simulink model "os_polyphase_synth_4096c"  (R2021a) (continued)
== system os_polyphase_synth_4096c/pfb_fir == (continued)
      | if( strcmp(use_hdl,'on')),
      |     set_param(gcb, 'use_embedded','off');
      |     set_param(gcb, 'MaskVisibilities',{'on','on','on','on','on','on','on','off'});
      | else,
      |     set_param(gcb, 'MaskVisibilities',{'on','on','on','on','on','on','on','on'});
      | end
  pol1_in1_tap94 : Subsystem  AncestorBlock=casper_library_pfbs/tap  mask(tap){mult_latency=2, coeff_width=18, coeff_frac_width=17, delay=512, data_width=18, bram_latency=1, use_hdl=off, use_embedded=on}
      mask prompts: mult_latency "Multiplier Latency"; coeff_width "Coefficient Bit Width In"; coeff_frac_width "Coefficient Fractional Bits"; delay "Data/Sync Delay"; data_width "Data Bit Width In"; bram_latency "BRAM Latency"; use_hdl "Use behavioral HDL for multlipliers"; use_embedded "Use embedded multipliers"
      mask init: same as os_polyphase_synth_4096c/pfb_fir/pol1_in1_tap1
      mask Callback use_hdl:
      | use_hdl = get_param(gcb,'use_hdl');
      | if( strcmp(use_hdl,'on')),
      |     set_param(gcb, 'use_embedded','off');
      |     set_param(gcb, 'MaskVisibilities',{'on','on','on','on','on','on','on','off'});
      | else,
      |     set_param(gcb, 'MaskVisibilities',{'on','on','on','on','on','on','on','on'});
      | end
  pol1_in1_tap95 : Subsystem  AncestorBlock=casper_library_pfbs/tap  mask(tap){mult_latency=2, coeff_width=18, coeff_frac_width=17, delay=512, data_width=18, bram_latency=1, use_hdl=off, use_embedded=on}
      mask prompts: mult_latency "Multiplier Latency"; coeff_width "Coefficient Bit Width In"; coeff_frac_width "Coefficient Fractional Bits"; delay "Data/Sync Delay"; data_width "Data Bit Width In"; bram_latency "BRAM Latency"; use_hdl "Use behavioral HDL for multlipliers"; use_embedded "Use embedded multipliers"
      mask init: same as os_polyphase_synth_4096c/pfb_fir/pol1_in1_tap1
      mask Callback use_hdl:
      | use_hdl = get_param(gcb,'use_hdl');
      | if( strcmp(use_hdl,'on')),
      |     set_param(gcb, 'use_embedded','off');
      |     set_param(gcb, 'MaskVisibilities',{'on','on','on','on','on','on','on','off'});
      | else,
      |     set_param(gcb, 'MaskVisibilities',{'on','on','on','on','on','on','on','on'});
      | end
  pol1_in1_tap96 : Subsystem  AncestorBlock=casper_library_pfbs/tap  mask(tap){mult_latency=2, coeff_width=18, coeff_frac_width=17, delay=512, data_width=18, bram_latency=1, use_hdl=off, use_embedded=on}
      mask prompts: mult_latency "Multiplier Latency"; coeff_width "Coefficient Bit Width In"; coeff_frac_width "Coefficient Fractional Bits"; delay "Data/Sync Delay"; data_width "Data Bit Width In"; bram_latency "BRAM Latency"; use_hdl "Use behavioral HDL for multlipliers"; use_embedded "Use embedded multipliers"
      mask init: same as os_polyphase_synth_4096c/pfb_fir/pol1_in1_tap1
      mask Callback use_hdl:
      | use_hdl = get_param(gcb,'use_hdl');
      | if( strcmp(use_hdl,'on')),
      |     set_param(gcb, 'use_embedded','off');
      |     set_param(gcb, 'MaskVisibilities',{'on','on','on','on','on','on','on','off'});
      | else,
      |     set_param(gcb, 'MaskVisibilities',{'on','on','on','on','on','on','on','on'});
      | end
  pol1_in1_tap97 : Subsystem  AncestorBlock=casper_library_pfbs/tap  mask(tap){mult_latency=2, coeff_width=18, coeff_frac_width=17, delay=512, data_width=18, bram_latency=1, use_hdl=off, use_embedded=on}
      mask prompts: mult_latency "Multiplier Latency"; coeff_width "Coefficient Bit Width In"; coeff_frac_width "Coefficient Fractional Bits"; delay "Data/Sync Delay"; data_width "Data Bit Width In"; bram_latency "BRAM Latency"; use_hdl "Use behavioral HDL for multlipliers"; use_embedded "Use embedded multipliers"
      mask init: same as os_polyphase_synth_4096c/pfb_fir/pol1_in1_tap1
      mask Callback use_hdl:
      | use_hdl = get_param(gcb,'use_hdl');
      | if( strcmp(use_hdl,'on')),
      |     set_param(gcb, 'use_embedded','off');
      |     set_param(gcb, 'MaskVisibilities',{'on','on','on','on','on','on','on','off'});
      | else,
      |     set_param(gcb, 'MaskVisibilities',{'on','on','on','on','on','on','on','on'});
      | end
  pol1_in1_tap98 : Subsystem  AncestorBlock=casper_library_pfbs/tap  mask(tap){mult_latency=2, coeff_width=18, coeff_frac_width=17, delay=512, data_width=18, bram_latency=1, use_hdl=off, use_embedded=on}
      mask prompts: mult_latency "Multiplier Latency"; coeff_width "Coefficient Bit Width In"; coeff_frac_width "Coefficient Fractional Bits"; delay "Data/Sync Delay"; data_width "Data Bit Width In"; bram_latency "BRAM Latency"; use_hdl "Use behavioral HDL for multlipliers"; use_embedded "Use embedded multipliers"
      mask init: same as os_polyphase_synth_4096c/pfb_fir/pol1_in1_tap1
      mask Callback use_hdl:
      | use_hdl = get_param(gcb,'use_hdl');
      | if( strcmp(use_hdl,'on')),
      |     set_param(gcb, 'use_embedded','off');
      |     set_param(gcb, 'MaskVisibilities',{'on','on','on','on','on','on','on','off'});
      | else,
      |     set_param(gcb, 'MaskVisibilities',{'on','on','on','on','on','on','on','on'});
      | end
  pol1_in1_tap99 : Subsystem  AncestorBlock=casper_library_pfbs/tap  mask(tap){mult_latency=2, coeff_width=18, coeff_frac_width=17, delay=512, data_width=18, bram_latency=1, use_hdl=off, use_embedded=on}
      mask prompts: mult_latency "Multiplier Latency"; coeff_width "Coefficient Bit Width In"; coeff_frac_width "Coefficient Fractional Bits"; delay "Data/Sync Delay"; data_width "Data Bit Width In"; bram_latency "BRAM Latency"; use_hdl "Use behavioral HDL for multlipliers"; use_embedded "Use embedded multipliers"
      mask init: same as os_polyphase_synth_4096c/pfb_fir/pol1_in1_tap1
      mask Callback use_hdl:
      | use_hdl = get_param(gcb,'use_hdl');
      | if( strcmp(use_hdl,'on')),
      |     set_param(gcb, 'use_embedded','off');
      |     set_param(gcb, 'MaskVisibilities',{'on','on','on','on','on','on','on','off'});
      | else,
      |     set_param(gcb, 'MaskVisibilities',{'on','on','on','on','on','on','on','on'});
      | end
  pol1_in2_coeffs : Subsystem  AncestorBlock=casper_library_pfbs/pfb_coeff_gen  mask(pfb_coeff_gen){PFBSize=12, CoeffBitWidth=18, TotalTaps=8, CoeffDistMem=off, WindowType=hamming, bram_latency=1, n_inputs=3, nput=1, fwidth=1, debug_mode=off, fan_latency=0}
      mask prompts: PFBSize "Size of PFB: (2^? pnts)"; CoeffBitWidth "Bitwidth of Coefficients:"; TotalTaps "Total Number of Taps:"; CoeffDistMem "Implement Coeff Gen in Distributed Memory"; WindowType "Windowing Function:"; bram_latency "BRAM Latency"; n_inputs "Number of Simultaneous Inputs: (2^?)"; nput "This is input number:"; fwidth "Bin Width (normal=1)"; debug_mode "Debug mode - coeffs are a [1:pfb_size*taps] ramp."; fan_latency "Fanout latency"
      mask init: same as os_polyphase_synth_4096c/pfb_fir/pol1_in1_coeffs
      settings: AttributesFormatString=|
      | PFBSize=12, n_inputs=3,
      | taps=8, input=1
  pol1_in3_coeffs : Subsystem  AncestorBlock=casper_library_pfbs/pfb_coeff_gen  mask(pfb_coeff_gen){PFBSize=12, CoeffBitWidth=18, TotalTaps=8, CoeffDistMem=off, WindowType=hamming, bram_latency=1, n_inputs=3, nput=2, fwidth=1, debug_mode=off, fan_latency=0}
      mask prompts: PFBSize "Size of PFB: (2^? pnts)"; CoeffBitWidth "Bitwidth of Coefficients:"; TotalTaps "Total Number of Taps:"; CoeffDistMem "Implement Coeff Gen in Distributed Memory"; WindowType "Windowing Function:"; bram_latency "BRAM Latency"; n_inputs "Number of Simultaneous Inputs: (2^?)"; nput "This is input number:"; fwidth "Bin Width (normal=1)"; debug_mode "Debug mode - coeffs are a [1:pfb_size*taps] ramp."; fan_latency "Fanout latency"
      mask init: same as os_polyphase_synth_4096c/pfb_fir/pol1_in1_coeffs
      settings: AttributesFormatString=|
      | PFBSize=12, n_inputs=3,
      | taps=8, input=2
  pol1_in4_coeffs : Subsystem  AncestorBlock=casper_library_pfbs/pfb_coeff_gen  mask(pfb_coeff_gen){PFBSize=12, CoeffBitWidth=18, TotalTaps=8, CoeffDistMem=off, WindowType=hamming, bram_latency=1, n_inputs=3, nput=3, fwidth=1, debug_mode=off, fan_latency=0}
      mask prompts: PFBSize "Size of PFB: (2^? pnts)"; CoeffBitWidth "Bitwidth of Coefficients:"; TotalTaps "Total Number of Taps:"; CoeffDistMem "Implement Coeff Gen in Distributed Memory"; WindowType "Windowing Function:"; bram_latency "BRAM Latency"; n_inputs "Number of Simultaneous Inputs: (2^?)"; nput "This is input number:"; fwidth "Bin Width (normal=1)"; debug_mode "Debug mode - coeffs are a [1:pfb_size*taps] ramp."; fan_latency "Fanout latency"
      mask init: same as os_polyphase_synth_4096c/pfb_fir/pol1_in1_coeffs
      settings: AttributesFormatString=|
      | PFBSize=12, n_inputs=3,
      | taps=8, input=3
  pol1_in5_coeffs : Subsystem  AncestorBlock=casper_library_pfbs/pfb_coeff_gen  mask(pfb_coeff_gen){PFBSize=12, CoeffBitWidth=18, TotalTaps=8, CoeffDistMem=off, WindowType=hamming, bram_latency=1, n_inputs=3, nput=4, fwidth=1, debug_mode=off, fan_latency=0}
      mask prompts: PFBSize "Size of PFB: (2^? pnts)"; CoeffBitWidth "Bitwidth of Coefficients:"; TotalTaps "Total Number of Taps:"; CoeffDistMem "Implement Coeff Gen in Distributed Memory"; WindowType "Windowing Function:"; bram_latency "BRAM Latency"; n_inputs "Number of Simultaneous Inputs: (2^?)"; nput "This is input number:"; fwidth "Bin Width (normal=1)"; debug_mode "Debug mode - coeffs are a [1:pfb_size*taps] ramp."; fan_latency "Fanout latency"
      mask init: same as os_polyphase_synth_4096c/pfb_fir/pol1_in1_coeffs
      settings: AttributesFormatString=|
      | PFBSize=12, n_inputs=3,
      | taps=8, input=4
  pol1_in6_coeffs : Subsystem  AncestorBlock=casper_library_pfbs/pfb_coeff_gen  mask(pfb_coeff_gen){PFBSize=12, CoeffBitWidth=18, TotalTaps=8, CoeffDistMem=off, WindowType=hamming, bram_latency=1, n_inputs=3, nput=5, fwidth=1, debug_mode=off, fan_latency=0}
      mask prompts: PFBSize "Size of PFB: (2^? pnts)"; CoeffBitWidth "Bitwidth of Coefficients:"; TotalTaps "Total Number of Taps:"; CoeffDistMem "Implement Coeff Gen in Distributed Memory"; WindowType "Windowing Function:"; bram_latency "BRAM Latency"; n_inputs "Number of Simultaneous Inputs: (2^?)"; nput "This is input number:"; fwidth "Bin Width (normal=1)"; debug_mode "Debug mode - coeffs are a [1:pfb_size*taps] ramp."; fan_latency "Fanout latency"
      mask init: same as os_polyphase_synth_4096c/pfb_fir/pol1_in1_coeffs
      settings: AttributesFormatString=|
      | PFBSize=12, n_inputs=3,
      | taps=8, input=5
  pol1_in7_coeffs : Subsystem  AncestorBlock=casper_library_pfbs/pfb_coeff_gen  mask(pfb_coeff_gen){PFBSize=12, CoeffBitWidth=18, TotalTaps=8, CoeffDistMem=off, WindowType=hamming, bram_latency=1, n_inputs=3, nput=6, fwidth=1, debug_mode=off, fan_latency=0}
      mask prompts: PFBSize "Size of PFB: (2^? pnts)"; CoeffBitWidth "Bitwidth of Coefficients:"; TotalTaps "Total Number of Taps:"; CoeffDistMem "Implement Coeff Gen in Distributed Memory"; WindowType "Windowing Function:"; bram_latency "BRAM Latency"; n_inputs "Number of Simultaneous Inputs: (2^?)"; nput "This is input number:"; fwidth "Bin Width (normal=1)"; debug_mode "Debug mode - coeffs are a [1:pfb_size*taps] ramp."; fan_latency "Fanout latency"
      mask init: same as os_polyphase_synth_4096c/pfb_fir/pol1_in1_coeffs
      settings: AttributesFormatString=|
      | PFBSize=12, n_inputs=3,
      | taps=8, input=6
  pol1_in8_coeffs : Subsystem  AncestorBlock=casper_library_pfbs/pfb_coeff_gen  mask(pfb_coeff_gen){PFBSize=12, CoeffBitWidth=18, TotalTaps=8, CoeffDistMem=off, WindowType=hamming, bram_latency=1, n_inputs=3, nput=7, fwidth=1, debug_mode=off, fan_latency=0}
      mask prompts: PFBSize "Size of PFB: (2^? pnts)"; CoeffBitWidth "Bitwidth of Coefficients:"; TotalTaps "Total Number of Taps:"; CoeffDistMem "Implement Coeff Gen in Distributed Memory"; WindowType "Windowing Function:"; bram_latency "BRAM Latency"; n_inputs "Number of Simultaneous Inputs: (2^?)"; nput "This is input number:"; fwidth "Bin Width (normal=1)"; debug_mode "Debug mode - coeffs are a [1:pfb_size*taps] ramp."; fan_latency "Fanout latency"
      mask init: same as os_polyphase_synth_4096c/pfb_fir/pol1_in1_coeffs
      settings: AttributesFormatString=|
      | PFBSize=12, n_inputs=3,
      | taps=8, input=7
  pol2_in1_delay : xbsIndex_r4/Delay  rst=off  infoeditControl=Selection of Reset will increase slice count due to use of real FFs and instead of SRLs  en=off  latency=bram_latency+fan_latency  reg_retiming=off  dbl_ovrd=off  block_type=delay
  pol2_in2_delay : xbsIndex_r4/Delay  rst=off  infoeditControl=Selection of Reset will increase slice count due to use of real FFs and instead of SRLs  en=off  latency=bram_latency+fan_latency  reg_retiming=off  dbl_ovrd=off  block_type=delay
  pol2_in3_delay : xbsIndex_r4/Delay  rst=off  infoeditControl=Selection of Reset will increase slice count due to use of real FFs and instead of SRLs  en=off  latency=bram_latency+fan_latency  reg_retiming=off  dbl_ovrd=off  block_type=delay
  pol2_in4_delay : xbsIndex_r4/Delay  rst=off  infoeditControl=Selection of Reset will increase slice count due to use of real FFs and instead of SRLs  en=off  latency=bram_latency+fan_latency  reg_retiming=off  dbl_ovrd=off  block_type=delay
  pol2_in5_delay : xbsIndex_r4/Delay  rst=off  infoeditControl=Selection of Reset will increase slice count due to use of real FFs and instead of SRLs  en=off  latency=bram_latency+fan_latency  reg_retiming=off  dbl_ovrd=off  block_type=delay
  pol2_in6_delay : xbsIndex_r4/Delay  rst=off  infoeditControl=Selection of Reset will increase slice count due to use of real FFs and instead of SRLs  en=off  latency=bram_latency+fan_latency  reg_retiming=off  dbl_ovrd=off  block_type=delay
  pol2_in7_delay : xbsIndex_r4/Delay  rst=off  infoeditControl=Selection of Reset will increase slice count due to use of real FFs and instead of SRLs  en=off  latency=bram_latency+fan_latency  reg_retiming=off  dbl_ovrd=off  block_type=delay
  pol2_in8_delay : xbsIndex_r4/Delay  rst=off  infoeditControl=Selection of Reset will increase slice count due to use of real FFs and instead of SRLs  en=off  latency=bram_latency+fan_latency  reg_retiming=off  dbl_ovrd=off  block_type=delay
  sync_out : Outport  VectorParamsAs1DForOutWhenUnconnected=off
  pol1_out1 : Outport  Port=2  VectorParamsAs1DForOutWhenUnconnected=off
  pol1_out2 : Outport  Port=3  VectorParamsAs1DForOutWhenUnconnected=off
  pol1_out3 : Outport  Port=4
  pol1_out4 : Outport  Port=5
  pol1_out5 : Outport  Port=6
  pol1_out6 : Outport  Port=7
  pol1_out7 : Outport  Port=8
  pol1_out8 : Outport  Port=9
  pol2_out1 : Outport  Port=10
  pol2_out2 : Outport  Port=11
  pol2_out3 : Outport  Port=12
  pol2_out4 : Outport  Port=13
  pol2_out5 : Outport  Port=14
  pol2_out6 : Outport  Port=15
  pol2_out7 : Outport  Port=16
  pol2_out8 : Outport  Port=17
  wires:
  pol1_in1/1 -> pol1_in1_coeffs/1(din)
  sync/1 -> pol1_in1_coeffs/2(sync), pol1_in2_coeffs/2(sync), pol1_in4_coeffs/2(sync), pol1_in3_coeffs/2(sync), pol1_in7_coeffs/2(sync), pol1_in6_coeffs/2(sync), pol1_in5_coeffs/2(sync), pol1_in8_coeffs/2(sync)
  pol1_in1_coeffs/1(dout) -> pol1_in1_first_tap/1(din)
  pol1_in1_coeffs/2(sync_out) -> pol1_in1_first_tap15/2(sync), pol1_in1_first_tap13/2(sync), pol1_in1_first_tap12/2(sync), pol1_in1_first_tap8/2(sync), pol1_in1_first_tap9/2(sync), pol1_in1_first_tap14/2(sync), pol1_in1_first_tap11/2(sync), pol1_in1_first_tap10/2(sync), pol1_in1_first_tap7/2(sync), pol1_in1_first_tap6/2(sync), pol1_in1_first_tap5/2(sync), pol1_in1_first_tap4/2(sync), pol1_in1_first_tap3/2(sync), pol1_in1_first_tap2/2(sync), pol1_in1_first_tap1/2(sync), pol1_in1_first_tap/2(sync)
  pol1_in1_coeffs/3(coeff) -> pol1_in1_first_tap8/3(coeffs), pol1_in1_first_tap/3(coeffs)
  pol1_in1_first_tap/1(dout) -> pol1_in1_tap2/1(din)
  pol1_in1_first_tap/2(sync_out) -> pol1_in1_tap2/2(sync)
  pol1_in1_first_tap/3(coeff_out) -> pol1_in1_tap2/3(coeff)
  pol1_in1_first_tap/4(taps_out) -> pol1_in1_tap2/4(taps)
  pol1_in1_tap2/1(dout) -> pol1_in1_tap1/1(din)
  pol1_in1_tap2/2(sync_out) -> pol1_in1_tap1/2(sync)
  pol1_in1_tap2/3(coeff_out) -> pol1_in1_tap1/3(coeff)
  pol1_in1_tap2/4(taps_out) -> pol1_in1_tap1/4(taps)
  pol1_in1_tap1/1(dout) -> pol1_in1_tap3/1(din)
  pol1_in1_tap1/2(sync_out) -> pol1_in1_tap3/2(sync)
  pol1_in1_tap1/3(coeff_out) -> pol1_in1_tap3/3(coeff)
  pol1_in1_tap1/4(taps_out) -> pol1_in1_tap3/4(taps)
  pol1_in1_tap3/1(dout) -> pol1_in1_tap4/1(din)
  pol1_in1_tap3/2(sync_out) -> pol1_in1_tap4/2(sync)
  pol1_in1_tap3/3(coeff_out) -> pol1_in1_tap4/3(coeff)
  pol1_in1_tap3/4(taps_out) -> pol1_in1_tap4/4(taps)
  pol1_in1_tap4/1(dout) -> pol1_in1_tap5/1(din)
  pol1_in1_tap4/2(sync_out) -> pol1_in1_tap5/2(sync)
  pol1_in1_tap4/3(coeff_out) -> pol1_in1_tap5/3(coeff)
  pol1_in1_tap4/4(taps_out) -> pol1_in1_tap5/4(taps)
  pol1_in1_tap5/1(dout) -> pol1_in1_tap6/1(din)
  pol1_in1_tap5/2(sync_out) -> pol1_in1_tap6/2(sync)
  pol1_in1_tap5/3(coeff_out) -> pol1_in1_tap6/3(coeff)
  pol1_in1_tap5/4(taps_out) -> pol1_in1_tap6/4(taps)
  pol1_in1_tap6/1(dout) -> pol1_in1_tap7/1(din)
  pol1_in1_tap6/2(sync_out) -> pol1_in1_tap7/2(sync)
  pol1_in1_tap6/3(coeff_out) -> pol1_in1_tap7/3(coeff)
  pol1_in1_tap6/4(taps_out) -> pol1_in1_tap7/4(taps)
  pol1_in2/1 -> pol1_in2_coeffs/1(din)
  pol1_in2_coeffs/1(dout) -> pol1_in1_first_tap1/1(din)
  pol1_in2_coeffs/3(coeff) -> pol1_in1_first_tap9/3(coeffs), pol1_in1_first_tap1/3(coeffs)
  pol1_in3/1 -> pol1_in3_coeffs/1(din)
  pol1_in3_coeffs/1(dout) -> pol1_in1_first_tap2/1(din)
  pol1_in3_coeffs/3(coeff) -> pol1_in1_first_tap10/3(coeffs), pol1_in1_first_tap2/3(coeffs)
  pol1_in4/1 -> pol1_in4_coeffs/1(din)
  pol1_in4_coeffs/1(dout) -> pol1_in1_first_tap3/1(din)
  pol1_in4_coeffs/3(coeff) -> pol1_in1_first_tap11/3(coeffs), pol1_in1_first_tap3/3(coeffs)
  pol1_in5/1 -> pol1_in5_coeffs/1(din)
  pol1_in5_coeffs/1(dout) -> pol1_in1_first_tap4/1(din)
  pol1_in5_coeffs/3(coeff) -> pol1_in1_first_tap12/3(coeffs), pol1_in1_first_tap4/3(coeffs)
  pol1_in6/1 -> pol1_in6_coeffs/1(din)
  pol1_in6_coeffs/1(dout) -> pol1_in1_first_tap5/1(din)
  pol1_in6_coeffs/3(coeff) -> pol1_in1_first_tap13/3(coeffs), pol1_in1_first_tap5/3(coeffs)
  pol1_in7/1 -> pol1_in7_coeffs/1(din)
  pol1_in7_coeffs/1(dout) -> pol1_in1_first_tap6/1(din)
  pol1_in7_coeffs/3(coeff) -> pol1_in1_first_tap14/3(coeffs), pol1_in1_first_tap6/3(coeffs)
  pol1_in8/1 -> pol1_in8_coeffs/1(din)
  pol1_in8_coeffs/1(dout) -> pol1_in1_first_tap7/1(din)
  pol1_in8_coeffs/3(coeff) -> pol1_in1_first_tap15/3(coeffs), pol1_in1_first_tap7/3(coeffs)
  pol2_in1/1 -> pol2_in1_delay/1
  pol2_in1_delay/1 -> pol1_in1_first_tap8/1(din)
  pol2_in2/1 -> pol2_in2_delay/1
  pol2_in2_delay/1 -> pol1_in1_first_tap9/1(din)
  pol2_in3/1 -> pol2_in3_delay/1
  pol2_in3_delay/1 -> pol1_in1_first_tap10/1(din)
  pol2_in4/1 -> pol2_in4_delay/1
  pol2_in4_delay/1 -> pol1_in1_first_tap11/1(din)
  pol2_in5/1 -> pol2_in5_delay/1
  pol2_in5_delay/1 -> pol1_in1_first_tap12/1(din)
  pol2_in6/1 -> pol2_in6_delay/1
  pol2_in6_delay/1 -> pol1_in1_first_tap13/1(din)
  pol2_in7/1 -> pol2_in7_delay/1
  pol2_in7_delay/1 -> pol1_in1_first_tap14/1(din)
  pol2_in8/1 -> pol2_in8_delay/1
  pol2_in8_delay/1 -> pol1_in1_first_tap15/1(din)
  pol1_in1_tap7/1(sync_out) -> sync_out/1
  pol1_in1_tap7/2(taps_out) -> pol1_out1/1
  pol1_in1_tap13/4(taps_out) -> pol1_in1_tap14/4(taps)
  pol1_in1_tap13/3(coeff_out) -> pol1_in1_tap14/3(coeff)
  pol1_in1_tap13/2(sync_out) -> pol1_in1_tap14/2(sync)
  pol1_in1_tap13/1(dout) -> pol1_in1_tap14/1(din)
  pol1_in1_tap12/4(taps_out) -> pol1_in1_tap13/4(taps)
  pol1_in1_tap12/3(coeff_out) -> pol1_in1_tap13/3(coeff)
  pol1_in1_tap12/2(sync_out) -> pol1_in1_tap13/2(sync)
  pol1_in1_tap12/1(dout) -> pol1_in1_tap13/1(din)
  pol1_in1_tap11/4(taps_out) -> pol1_in1_tap12/4(taps)
  pol1_in1_tap11/3(coeff_out) -> pol1_in1_tap12/3(coeff)
  pol1_in1_tap11/2(sync_out) -> pol1_in1_tap12/2(sync)
  pol1_in1_tap11/1(dout) -> pol1_in1_tap12/1(din)
  pol1_in1_tap10/4(taps_out) -> pol1_in1_tap11/4(taps)
  pol1_in1_tap10/3(coeff_out) -> pol1_in1_tap11/3(coeff)
  pol1_in1_tap10/2(sync_out) -> pol1_in1_tap11/2(sync)
  pol1_in1_tap10/1(dout) -> pol1_in1_tap11/1(din)
  pol1_in1_tap8/4(taps_out) -> pol1_in1_tap10/4(taps)
  pol1_in1_tap8/3(coeff_out) -> pol1_in1_tap10/3(coeff)
  pol1_in1_tap8/2(sync_out) -> pol1_in1_tap10/2(sync)
  pol1_in1_tap8/1(dout) -> pol1_in1_tap10/1(din)
  pol1_in1_tap9/4(taps_out) -> pol1_in1_tap8/4(taps)
  pol1_in1_tap9/3(coeff_out) -> pol1_in1_tap8/3(coeff)
  pol1_in1_tap9/2(sync_out) -> pol1_in1_tap8/2(sync)
  pol1_in1_tap9/1(dout) -> pol1_in1_tap8/1(din)
  pol1_in1_first_tap1/4(taps_out) -> pol1_in1_tap9/4(taps)
  pol1_in1_first_tap1/3(coeff_out) -> pol1_in1_tap9/3(coeff)
  pol1_in1_first_tap1/2(sync_out) -> pol1_in1_tap9/2(sync)
  pol1_in1_first_tap1/1(dout) -> pol1_in1_tap9/1(din)
  pol1_in1_first_tap2/1(dout) -> pol1_in1_tap21/1(din)
  pol1_in1_first_tap2/2(sync_out) -> pol1_in1_tap21/2(sync)
  pol1_in1_first_tap2/3(coeff_out) -> pol1_in1_tap21/3(coeff)
  pol1_in1_first_tap2/4(taps_out) -> pol1_in1_tap21/4(taps)
  pol1_in1_tap21/1(dout) -> pol1_in1_tap20/1(din)
  pol1_in1_tap21/2(sync_out) -> pol1_in1_tap20/2(sync)
  pol1_in1_tap21/3(coeff_out) -> pol1_in1_tap20/3(coeff)
  pol1_in1_tap21/4(taps_out) -> pol1_in1_tap20/4(taps)
  pol1_in1_tap20/1(dout) -> pol1_in1_tap15/1(din)
  pol1_in1_tap20/2(sync_out) -> pol1_in1_tap15/2(sync)
  pol1_in1_tap20/3(coeff_out) -> pol1_in1_tap15/3(coeff)
  pol1_in1_tap20/4(taps_out) -> pol1_in1_tap15/4(taps)
  pol1_in1_tap15/1(dout) -> pol1_in1_tap16/1(din)
  pol1_in1_tap15/2(sync_out) -> pol1_in1_tap16/2(sync)
  pol1_in1_tap15/3(coeff_out) -> pol1_in1_tap16/3(coeff)
  pol1_in1_tap15/4(taps_out) -> pol1_in1_tap16/4(taps)
  pol1_in1_tap16/1(dout) -> pol1_in1_tap17/1(din)
  pol1_in1_tap16/2(sync_out) -> pol1_in1_tap17/2(sync)
  pol1_in1_tap16/3(coeff_out) -> pol1_in1_tap17/3(coeff)
  pol1_in1_tap16/4(taps_out) -> pol1_in1_tap17/4(taps)
  pol1_in1_tap17/1(dout) -> pol1_in1_tap18/1(din)
  pol1_in1_tap17/2(sync_out) -> pol1_in1_tap18/2(sync)
  pol1_in1_tap17/3(coeff_out) -> pol1_in1_tap18/3(coeff)
  pol1_in1_tap17/4(taps_out) -> pol1_in1_tap18/4(taps)
  pol1_in1_tap18/1(dout) -> pol1_in1_tap19/1(din)
  pol1_in1_tap18/2(sync_out) -> pol1_in1_tap19/2(sync)
  pol1_in1_tap18/3(coeff_out) -> pol1_in1_tap19/3(coeff)
  pol1_in1_tap18/4(taps_out) -> pol1_in1_tap19/4(taps)
  pol1_in1_tap25/4(taps_out) -> pol1_in1_tap26/4(taps)
  pol1_in1_tap25/3(coeff_out) -> pol1_in1_tap26/3(coeff)
  pol1_in1_tap25/2(sync_out) -> pol1_in1_tap26/2(sync)
  pol1_in1_tap25/1(dout) -> pol1_in1_tap26/1(din)
  pol1_in1_tap24/4(taps_out) -> pol1_in1_tap25/4(taps)
  pol1_in1_tap24/3(coeff_out) -> pol1_in1_tap25/3(coeff)
  pol1_in1_tap24/2(sync_out) -> pol1_in1_tap25/2(sync)
  pol1_in1_tap24/1(dout) -> pol1_in1_tap25/1(din)
  pol1_in1_tap23/4(taps_out) -> pol1_in1_tap24/4(taps)
  pol1_in1_tap23/3(coeff_out) -> pol1_in1_tap24/3(coeff)
  pol1_in1_tap23/2(sync_out) -> pol1_in1_tap24/2(sync)
  pol1_in1_tap23/1(dout) -> pol1_in1_tap24/1(din)
  pol1_in1_tap22/4(taps_out) -> pol1_in1_tap23/4(taps)
  pol1_in1_tap22/3(coeff_out) -> pol1_in1_tap23/3(coeff)
  pol1_in1_tap22/2(sync_out) -> pol1_in1_tap23/2(sync)
  pol1_in1_tap22/1(dout) -> pol1_in1_tap23/1(din)
  pol1_in1_tap27/4(taps_out) -> pol1_in1_tap22/4(taps)
  pol1_in1_tap27/3(coeff_out) -> pol1_in1_tap22/3(coeff)
  pol1_in1_tap27/2(sync_out) -> pol1_in1_tap22/2(sync)
  pol1_in1_tap27/1(dout) -> pol1_in1_tap22/1(din)
  pol1_in1_tap28/4(taps_out) -> pol1_in1_tap27/4(taps)
  pol1_in1_tap28/3(coeff_out) -> pol1_in1_tap27/3(coeff)
  pol1_in1_tap28/2(sync_out) -> pol1_in1_tap27/2(sync)
  pol1_in1_tap28/1(dout) -> pol1_in1_tap27/1(din)
  pol1_in1_first_tap3/4(taps_out) -> pol1_in1_tap28/4(taps)
  pol1_in1_first_tap3/3(coeff_out) -> pol1_in1_tap28/3(coeff)
  pol1_in1_first_tap3/2(sync_out) -> pol1_in1_tap28/2(sync)
  pol1_in1_first_tap3/1(dout) -> pol1_in1_tap28/1(din)
  pol1_in1_first_tap7/1(dout) -> pol1_in1_tap49/1(din)
  pol1_in1_first_tap7/2(sync_out) -> pol1_in1_tap49/2(sync)
  pol1_in1_first_tap7/3(coeff_out) -> pol1_in1_tap49/3(coeff)
  pol1_in1_first_tap7/4(taps_out) -> pol1_in1_tap49/4(taps)
  pol1_in1_tap49/1(dout) -> pol1_in1_tap48/1(din)
  pol1_in1_tap49/2(sync_out) -> pol1_in1_tap48/2(sync)
  pol1_in1_tap49/3(coeff_out) -> pol1_in1_tap48/3(coeff)
  pol1_in1_tap49/4(taps_out) -> pol1_in1_tap48/4(taps)
  pol1_in1_tap48/1(dout) -> pol1_in1_tap43/1(din)
  pol1_in1_tap48/2(sync_out) -> pol1_in1_tap43/2(sync)
  pol1_in1_tap48/3(coeff_out) -> pol1_in1_tap43/3(coeff)
  pol1_in1_tap48/4(taps_out) -> pol1_in1_tap43/4(taps)
  pol1_in1_tap43/1(dout) -> pol1_in1_tap44/1(din)
  pol1_in1_tap43/2(sync_out) -> pol1_in1_tap44/2(sync)
  pol1_in1_tap43/3(coeff_out) -> pol1_in1_tap44/3(coeff)
  pol1_in1_tap43/4(taps_out) -> pol1_in1_tap44/4(taps)
  pol1_in1_tap44/1(dout) -> pol1_in1_tap45/1(din)
  pol1_in1_tap44/2(sync_out) -> pol1_in1_tap45/2(sync)
  pol1_in1_tap44/3(coeff_out) -> pol1_in1_tap45/3(coeff)
  pol1_in1_tap44/4(taps_out) -> pol1_in1_tap45/4(taps)
  pol1_in1_tap45/1(dout) -> pol1_in1_tap46/1(din)
  pol1_in1_tap45/2(sync_out) -> pol1_in1_tap46/2(sync)
  pol1_in1_tap45/3(coeff_out) -> pol1_in1_tap46/3(coeff)
  pol1_in1_tap45/4(taps_out) -> pol1_in1_tap46/4(taps)
  pol1_in1_tap46/1(dout) -> pol1_in1_tap47/1(din)
  pol1_in1_tap46/2(sync_out) -> pol1_in1_tap47/2(sync)
  pol1_in1_tap46/3(coeff_out) -> pol1_in1_tap47/3(coeff)
  pol1_in1_tap46/4(taps_out) -> pol1_in1_tap47/4(taps)
  pol1_in1_tap38/4(taps_out) -> pol1_in1_tap39/4(taps)
  pol1_in1_tap38/3(coeff_out) -> pol1_in1_tap39/3(coeff)
  pol1_in1_tap38/2(sync_out) -> pol1_in1_tap39/2(sync)
  pol1_in1_tap38/1(dout) -> pol1_in1_tap39/1(din)
  pol1_in1_tap37/4(taps_out) -> pol1_in1_tap38/4(taps)
  pol1_in1_tap37/3(coeff_out) -> pol1_in1_tap38/3(coeff)
  pol1_in1_tap37/2(sync_out) -> pol1_in1_tap38/2(sync)
  pol1_in1_tap37/1(dout) -> pol1_in1_tap38/1(din)
  pol1_in1_tap36/4(taps_out) -> pol1_in1_tap37/4(taps)
  pol1_in1_tap36/3(coeff_out) -> pol1_in1_tap37/3(coeff)
  pol1_in1_tap36/2(sync_out) -> pol1_in1_tap37/2(sync)
  pol1_in1_tap36/1(dout) -> pol1_in1_tap37/1(din)
  pol1_in1_tap35/4(taps_out) -> pol1_in1_tap36/4(taps)
  pol1_in1_tap35/3(coeff_out) -> pol1_in1_tap36/3(coeff)
  pol1_in1_tap35/2(sync_out) -> pol1_in1_tap36/2(sync)
  pol1_in1_tap35/1(dout) -> pol1_in1_tap36/1(din)
  pol1_in1_tap41/4(taps_out) -> pol1_in1_tap35/4(taps)
  pol1_in1_tap41/3(coeff_out) -> pol1_in1_tap35/3(coeff)
  pol1_in1_tap41/2(sync_out) -> pol1_in1_tap35/2(sync)
  pol1_in1_tap41/1(dout) -> pol1_in1_tap35/1(din)
  pol1_in1_tap42/4(taps_out) -> pol1_in1_tap41/4(taps)
  pol1_in1_tap42/3(coeff_out) -> pol1_in1_tap41/3(coeff)
  pol1_in1_tap42/2(sync_out) -> pol1_in1_tap41/2(sync)
  pol1_in1_tap42/1(dout) -> pol1_in1_tap41/1(din)
  pol1_in1_first_tap6/4(taps_out) -> pol1_in1_tap42/4(taps)
  pol1_in1_first_tap6/3(coeff_out) -> pol1_in1_tap42/3(coeff)
  pol1_in1_first_tap6/2(sync_out) -> pol1_in1_tap42/2(sync)
  pol1_in1_first_tap6/1(dout) -> pol1_in1_tap42/1(din)
  pol1_in1_first_tap5/1(dout) -> pol1_in1_tap56/1(din)
  pol1_in1_first_tap5/2(sync_out) -> pol1_in1_tap56/2(sync)
  pol1_in1_first_tap5/3(coeff_out) -> pol1_in1_tap56/3(coeff)
  pol1_in1_first_tap5/4(taps_out) -> pol1_in1_tap56/4(taps)
  pol1_in1_tap56/1(dout) -> pol1_in1_tap55/1(din)
  pol1_in1_tap56/2(sync_out) -> pol1_in1_tap55/2(sync)
  pol1_in1_tap56/3(coeff_out) -> pol1_in1_tap55/3(coeff)
  pol1_in1_tap56/4(taps_out) -> pol1_in1_tap55/4(taps)
  pol1_in1_tap55/1(dout) -> pol1_in1_tap30/1(din)
  pol1_in1_tap55/2(sync_out) -> pol1_in1_tap30/2(sync)
  pol1_in1_tap55/3(coeff_out) -> pol1_in1_tap30/3(coeff)
  pol1_in1_tap55/4(taps_out) -> pol1_in1_tap30/4(taps)
  pol1_in1_tap30/1(dout) -> pol1_in1_tap31/1(din)
  pol1_in1_tap30/2(sync_out) -> pol1_in1_tap31/2(sync)
  pol1_in1_tap30/3(coeff_out) -> pol1_in1_tap31/3(coeff)
  pol1_in1_tap30/4(taps_out) -> pol1_in1_tap31/4(taps)
  pol1_in1_tap31/1(dout) -> pol1_in1_tap32/1(din)
  pol1_in1_tap31/2(sync_out) -> pol1_in1_tap32/2(sync)
  pol1_in1_tap31/3(coeff_out) -> pol1_in1_tap32/3(coeff)
  pol1_in1_tap31/4(taps_out) -> pol1_in1_tap32/4(taps)
  pol1_in1_tap32/1(dout) -> pol1_in1_tap33/1(din)
  pol1_in1_tap32/2(sync_out) -> pol1_in1_tap33/2(sync)
  pol1_in1_tap32/3(coeff_out) -> pol1_in1_tap33/3(coeff)
  pol1_in1_tap32/4(taps_out) -> pol1_in1_tap33/4(taps)
  pol1_in1_tap33/1(dout) -> pol1_in1_tap34/1(din)
  pol1_in1_tap33/2(sync_out) -> pol1_in1_tap34/2(sync)
  pol1_in1_tap33/3(coeff_out) -> pol1_in1_tap34/3(coeff)
  pol1_in1_tap33/4(taps_out) -> pol1_in1_tap34/4(taps)
  pol1_in1_tap53/4(taps_out) -> pol1_in1_tap54/4(taps)
  pol1_in1_tap53/3(coeff_out) -> pol1_in1_tap54/3(coeff)
  pol1_in1_tap53/2(sync_out) -> pol1_in1_tap54/2(sync)
  pol1_in1_tap53/1(dout) -> pol1_in1_tap54/1(din)
  pol1_in1_tap52/4(taps_out) -> pol1_in1_tap53/4(taps)
  pol1_in1_tap52/3(coeff_out) -> pol1_in1_tap53/3(coeff)
  pol1_in1_tap52/2(sync_out) -> pol1_in1_tap53/2(sync)
  pol1_in1_tap52/1(dout) -> pol1_in1_tap53/1(din)
  pol1_in1_tap51/4(taps_out) -> pol1_in1_tap52/4(taps)
  pol1_in1_tap51/3(coeff_out) -> pol1_in1_tap52/3(coeff)
  pol1_in1_tap51/2(sync_out) -> pol1_in1_tap52/2(sync)
  pol1_in1_tap51/1(dout) -> pol1_in1_tap52/1(din)
  pol1_in1_tap50/4(taps_out) -> pol1_in1_tap51/4(taps)
  pol1_in1_tap50/3(coeff_out) -> pol1_in1_tap51/3(coeff)
  pol1_in1_tap50/2(sync_out) -> pol1_in1_tap51/2(sync)
  pol1_in1_tap50/1(dout) -> pol1_in1_tap51/1(din)
  pol1_in1_tap29/4(taps_out) -> pol1_in1_tap50/4(taps)
  pol1_in1_tap29/3(coeff_out) -> pol1_in1_tap50/3(coeff)
  pol1_in1_tap29/2(sync_out) -> pol1_in1_tap50/2(sync)
  pol1_in1_tap29/1(dout) -> pol1_in1_tap50/1(din)
  pol1_in1_tap40/4(taps_out) -> pol1_in1_tap29/4(taps)
  pol1_in1_tap40/3(coeff_out) -> pol1_in1_tap29/3(coeff)
  pol1_in1_tap40/2(sync_out) -> pol1_in1_tap29/2(sync)
  pol1_in1_tap40/1(dout) -> pol1_in1_tap29/1(din)
  pol1_in1_first_tap4/4(taps_out) -> pol1_in1_tap40/4(taps)
  pol1_in1_first_tap4/3(coeff_out) -> pol1_in1_tap40/3(coeff)
  pol1_in1_first_tap4/2(sync_out) -> pol1_in1_tap40/2(sync)
  pol1_in1_first_tap4/1(dout) -> pol1_in1_tap40/1(din)
  pol1_in1_first_tap12/1(dout) -> pol1_in1_tap91/1(din)
  pol1_in1_first_tap12/2(sync_out) -> pol1_in1_tap91/2(sync)
  pol1_in1_first_tap12/3(coeff_out) -> pol1_in1_tap91/3(coeff)
  pol1_in1_first_tap12/4(taps_out) -> pol1_in1_tap91/4(taps)
  pol1_in1_tap91/1(dout) -> pol1_in1_tap78/1(din)
  pol1_in1_tap91/2(sync_out) -> pol1_in1_tap78/2(sync)
  pol1_in1_tap91/3(coeff_out) -> pol1_in1_tap78/3(coeff)
  pol1_in1_tap91/4(taps_out) -> pol1_in1_tap78/4(taps)
  pol1_in1_tap78/1(dout) -> pol1_in1_tap102/1(din)
  pol1_in1_tap78/2(sync_out) -> pol1_in1_tap102/2(sync)
  pol1_in1_tap78/3(coeff_out) -> pol1_in1_tap102/3(coeff)
  pol1_in1_tap78/4(taps_out) -> pol1_in1_tap102/4(taps)
  pol1_in1_tap102/1(dout) -> pol1_in1_tap103/1(din)
  pol1_in1_tap102/2(sync_out) -> pol1_in1_tap103/2(sync)
  pol1_in1_tap102/3(coeff_out) -> pol1_in1_tap103/3(coeff)
  pol1_in1_tap102/4(taps_out) -> pol1_in1_tap103/4(taps)
  pol1_in1_tap103/1(dout) -> pol1_in1_tap104/1(din)
  pol1_in1_tap103/2(sync_out) -> pol1_in1_tap104/2(sync)
  pol1_in1_tap103/3(coeff_out) -> pol1_in1_tap104/3(coeff)
  pol1_in1_tap103/4(taps_out) -> pol1_in1_tap104/4(taps)
  pol1_in1_tap104/1(dout) -> pol1_in1_tap105/1(din)
  pol1_in1_tap104/2(sync_out) -> pol1_in1_tap105/2(sync)
  pol1_in1_tap104/3(coeff_out) -> pol1_in1_tap105/3(coeff)
  pol1_in1_tap104/4(taps_out) -> pol1_in1_tap105/4(taps)
  pol1_in1_tap105/1(dout) -> pol1_in1_tap106/1(din)
  pol1_in1_tap105/2(sync_out) -> pol1_in1_tap106/2(sync)
  pol1_in1_tap105/3(coeff_out) -> pol1_in1_tap106/3(coeff)
  pol1_in1_tap105/4(taps_out) -> pol1_in1_tap106/4(taps)
  pol1_in1_tap83/4(taps_out) -> pol1_in1_tap84/4(taps)
  pol1_in1_tap83/3(coeff_out) -> pol1_in1_tap84/3(coeff)
  pol1_in1_tap83/2(sync_out) -> pol1_in1_tap84/2(sync)
  pol1_in1_tap83/1(dout) -> pol1_in1_tap84/1(din)
  pol1_in1_tap82/4(taps_out) -> pol1_in1_tap83/4(taps)
  pol1_in1_tap82/3(coeff_out) -> pol1_in1_tap83/3(coeff)
  pol1_in1_tap82/2(sync_out) -> pol1_in1_tap83/2(sync)
  pol1_in1_tap82/1(dout) -> pol1_in1_tap83/1(din)
  pol1_in1_tap81/4(taps_out) -> pol1_in1_tap82/4(taps)
  pol1_in1_tap81/3(coeff_out) -> pol1_in1_tap82/3(coeff)
  pol1_in1_tap81/2(sync_out) -> pol1_in1_tap82/2(sync)
  pol1_in1_tap81/1(dout) -> pol1_in1_tap82/1(din)
  pol1_in1_tap80/4(taps_out) -> pol1_in1_tap81/4(taps)
  pol1_in1_tap80/3(coeff_out) -> pol1_in1_tap81/3(coeff)
  pol1_in1_tap80/2(sync_out) -> pol1_in1_tap81/2(sync)
  pol1_in1_tap80/1(dout) -> pol1_in1_tap81/1(din)
  pol1_in1_tap107/4(taps_out) -> pol1_in1_tap80/4(taps)
  pol1_in1_tap107/3(coeff_out) -> pol1_in1_tap80/3(coeff)
  pol1_in1_tap107/2(sync_out) -> pol1_in1_tap80/2(sync)
  pol1_in1_tap107/1(dout) -> pol1_in1_tap80/1(din)
  pol1_in1_tap108/4(taps_out) -> pol1_in1_tap107/4(taps)
  pol1_in1_tap108/3(coeff_out) -> pol1_in1_tap107/3(coeff)
  pol1_in1_tap108/2(sync_out) -> pol1_in1_tap107/2(sync)
  pol1_in1_tap108/1(dout) -> pol1_in1_tap107/1(din)
  pol1_in1_first_tap13/4(taps_out) -> pol1_in1_tap108/4(taps)
  pol1_in1_first_tap13/3(coeff_out) -> pol1_in1_tap108/3(coeff)
  pol1_in1_first_tap13/2(sync_out) -> pol1_in1_tap108/2(sync)
  pol1_in1_first_tap13/1(dout) -> pol1_in1_tap108/1(din)
  pol1_in1_first_tap14/1(dout) -> pol1_in1_tap93/1(din)
  pol1_in1_first_tap14/2(sync_out) -> pol1_in1_tap93/2(sync)
  pol1_in1_first_tap14/3(coeff_out) -> pol1_in1_tap93/3(coeff)
  pol1_in1_first_tap14/4(taps_out) -> pol1_in1_tap93/4(taps)
  pol1_in1_tap93/1(dout) -> pol1_in1_tap92/1(din)
  pol1_in1_tap93/2(sync_out) -> pol1_in1_tap92/2(sync)
  pol1_in1_tap93/3(coeff_out) -> pol1_in1_tap92/3(coeff)
  pol1_in1_tap93/4(taps_out) -> pol1_in1_tap92/4(taps)
  pol1_in1_tap92/1(dout) -> pol1_in1_tap85/1(din)
  pol1_in1_tap92/2(sync_out) -> pol1_in1_tap85/2(sync)
  pol1_in1_tap92/3(coeff_out) -> pol1_in1_tap85/3(coeff)
  pol1_in1_tap92/4(taps_out) -> pol1_in1_tap85/4(taps)
  pol1_in1_tap85/1(dout) -> pol1_in1_tap86/1(din)
  pol1_in1_tap85/2(sync_out) -> pol1_in1_tap86/2(sync)
  pol1_in1_tap85/3(coeff_out) -> pol1_in1_tap86/3(coeff)
  pol1_in1_tap85/4(taps_out) -> pol1_in1_tap86/4(taps)
  pol1_in1_tap86/1(dout) -> pol1_in1_tap87/1(din)
  pol1_in1_tap86/2(sync_out) -> pol1_in1_tap87/2(sync)
  pol1_in1_tap86/3(coeff_out) -> pol1_in1_tap87/3(coeff)
  pol1_in1_tap86/4(taps_out) -> pol1_in1_tap87/4(taps)
  pol1_in1_tap87/1(dout) -> pol1_in1_tap88/1(din)
  pol1_in1_tap87/2(sync_out) -> pol1_in1_tap88/2(sync)
  pol1_in1_tap87/3(coeff_out) -> pol1_in1_tap88/3(coeff)
  pol1_in1_tap87/4(taps_out) -> pol1_in1_tap88/4(taps)
  pol1_in1_tap88/1(dout) -> pol1_in1_tap89/1(din)
  pol1_in1_tap88/2(sync_out) -> pol1_in1_tap89/2(sync)
  pol1_in1_tap88/3(coeff_out) -> pol1_in1_tap89/3(coeff)
  pol1_in1_tap88/4(taps_out) -> pol1_in1_tap89/4(taps)
  pol1_in1_tap97/4(taps_out) -> pol1_in1_tap98/4(taps)
  pol1_in1_tap97/3(coeff_out) -> pol1_in1_tap98/3(coeff)
  pol1_in1_tap97/2(sync_out) -> pol1_in1_tap98/2(sync)
  pol1_in1_tap97/1(dout) -> pol1_in1_tap98/1(din)
  pol1_in1_tap96/4(taps_out) -> pol1_in1_tap97/4(taps)
  pol1_in1_tap96/3(coeff_out) -> pol1_in1_tap97/3(coeff)
  pol1_in1_tap96/2(sync_out) -> pol1_in1_tap97/2(sync)
  pol1_in1_tap96/1(dout) -> pol1_in1_tap97/1(din)
  pol1_in1_tap95/4(taps_out) -> pol1_in1_tap96/4(taps)
  pol1_in1_tap95/3(coeff_out) -> pol1_in1_tap96/3(coeff)
  pol1_in1_tap95/2(sync_out) -> pol1_in1_tap96/2(sync)
  pol1_in1_tap95/1(dout) -> pol1_in1_tap96/1(din)
  pol1_in1_tap94/4(taps_out) -> pol1_in1_tap95/4(taps)
  pol1_in1_tap94/3(coeff_out) -> pol1_in1_tap95/3(coeff)
  pol1_in1_tap94/2(sync_out) -> pol1_in1_tap95/2(sync)
  pol1_in1_tap94/1(dout) -> pol1_in1_tap95/1(din)
  pol1_in1_tap99/4(taps_out) -> pol1_in1_tap94/4(taps)
  pol1_in1_tap99/3(coeff_out) -> pol1_in1_tap94/3(coeff)
  pol1_in1_tap99/2(sync_out) -> pol1_in1_tap94/2(sync)
  pol1_in1_tap99/1(dout) -> pol1_in1_tap94/1(din)
  pol1_in1_tap100/4(taps_out) -> pol1_in1_tap99/4(taps)
  pol1_in1_tap100/3(coeff_out) -> pol1_in1_tap99/3(coeff)
  pol1_in1_tap100/2(sync_out) -> pol1_in1_tap99/2(sync)
  pol1_in1_tap100/1(dout) -> pol1_in1_tap99/1(din)
  pol1_in1_first_tap15/4(taps_out) -> pol1_in1_tap100/4(taps)
  pol1_in1_first_tap15/3(coeff_out) -> pol1_in1_tap100/3(coeff)
  pol1_in1_first_tap15/2(sync_out) -> pol1_in1_tap100/2(sync)
  pol1_in1_first_tap15/1(dout) -> pol1_in1_tap100/1(din)
  pol1_in1_first_tap11/1(dout) -> pol1_in1_tap77/1(din)
  pol1_in1_first_tap11/2(sync_out) -> pol1_in1_tap77/2(sync)
  pol1_in1_first_tap11/3(coeff_out) -> pol1_in1_tap77/3(coeff)
  pol1_in1_first_tap11/4(taps_out) -> pol1_in1_tap77/4(taps)
  pol1_in1_tap77/1(dout) -> pol1_in1_tap76/1(din)
  pol1_in1_tap77/2(sync_out) -> pol1_in1_tap76/2(sync)
  pol1_in1_tap77/3(coeff_out) -> pol1_in1_tap76/3(coeff)
  pol1_in1_tap77/4(taps_out) -> pol1_in1_tap76/4(taps)
  pol1_in1_tap76/1(dout) -> pol1_in1_tap71/1(din)
  pol1_in1_tap76/2(sync_out) -> pol1_in1_tap71/2(sync)
  pol1_in1_tap76/3(coeff_out) -> pol1_in1_tap71/3(coeff)
  pol1_in1_tap76/4(taps_out) -> pol1_in1_tap71/4(taps)
  pol1_in1_tap71/1(dout) -> pol1_in1_tap72/1(din)
  pol1_in1_tap71/2(sync_out) -> pol1_in1_tap72/2(sync)
  pol1_in1_tap71/3(coeff_out) -> pol1_in1_tap72/3(coeff)
  pol1_in1_tap71/4(taps_out) -> pol1_in1_tap72/4(taps)
  pol1_in1_tap72/1(dout) -> pol1_in1_tap73/1(din)
  pol1_in1_tap72/2(sync_out) -> pol1_in1_tap73/2(sync)
  pol1_in1_tap72/3(coeff_out) -> pol1_in1_tap73/3(coeff)
  pol1_in1_tap72/4(taps_out) -> pol1_in1_tap73/4(taps)
  pol1_in1_tap73/1(dout) -> pol1_in1_tap74/1(din)
  pol1_in1_tap73/2(sync_out) -> pol1_in1_tap74/2(sync)
  pol1_in1_tap73/3(coeff_out) -> pol1_in1_tap74/3(coeff)
  pol1_in1_tap73/4(taps_out) -> pol1_in1_tap74/4(taps)
  pol1_in1_tap74/1(dout) -> pol1_in1_tap75/1(din)
  pol1_in1_tap74/2(sync_out) -> pol1_in1_tap75/2(sync)
  pol1_in1_tap74/3(coeff_out) -> pol1_in1_tap75/3(coeff)
  pol1_in1_tap74/4(taps_out) -> pol1_in1_tap75/4(taps)
  pol1_in1_tap66/4(taps_out) -> pol1_in1_tap67/4(taps)
  pol1_in1_tap66/3(coeff_out) -> pol1_in1_tap67/3(coeff)
  pol1_in1_tap66/2(sync_out) -> pol1_in1_tap67/2(sync)
  pol1_in1_tap66/1(dout) -> pol1_in1_tap67/1(din)
  pol1_in1_tap65/4(taps_out) -> pol1_in1_tap66/4(taps)
  pol1_in1_tap65/3(coeff_out) -> pol1_in1_tap66/3(coeff)
  pol1_in1_tap65/2(sync_out) -> pol1_in1_tap66/2(sync)
  pol1_in1_tap65/1(dout) -> pol1_in1_tap66/1(din)
  pol1_in1_tap64/4(taps_out) -> pol1_in1_tap65/4(taps)
  pol1_in1_tap64/3(coeff_out) -> pol1_in1_tap65/3(coeff)
  pol1_in1_tap64/2(sync_out) -> pol1_in1_tap65/2(sync)
  pol1_in1_tap64/1(dout) -> pol1_in1_tap65/1(din)
  pol1_in1_tap63/4(taps_out) -> pol1_in1_tap64/4(taps)
  pol1_in1_tap63/3(coeff_out) -> pol1_in1_tap64/3(coeff)
  pol1_in1_tap63/2(sync_out) -> pol1_in1_tap64/2(sync)
  pol1_in1_tap63/1(dout) -> pol1_in1_tap64/1(din)
  pol1_in1_tap69/4(taps_out) -> pol1_in1_tap63/4(taps)
  pol1_in1_tap69/3(coeff_out) -> pol1_in1_tap63/3(coeff)
  pol1_in1_tap69/2(sync_out) -> pol1_in1_tap63/2(sync)
  pol1_in1_tap69/1(dout) -> pol1_in1_tap63/1(din)
  pol1_in1_tap70/4(taps_out) -> pol1_in1_tap69/4(taps)
  pol1_in1_tap70/3(coeff_out) -> pol1_in1_tap69/3(coeff)
  pol1_in1_tap70/2(sync_out) -> pol1_in1_tap69/2(sync)
  pol1_in1_tap70/1(dout) -> pol1_in1_tap69/1(din)
  pol1_in1_first_tap10/4(taps_out) -> pol1_in1_tap70/4(taps)
  pol1_in1_first_tap10/3(coeff_out) -> pol1_in1_tap70/3(coeff)
  pol1_in1_first_tap10/2(sync_out) -> pol1_in1_tap70/2(sync)
  pol1_in1_first_tap10/1(dout) -> pol1_in1_tap70/1(din)
  pol1_in1_first_tap9/1(dout) -> pol1_in1_tap112/1(din)
  pol1_in1_first_tap9/2(sync_out) -> pol1_in1_tap112/2(sync)
  pol1_in1_first_tap9/3(coeff_out) -> pol1_in1_tap112/3(coeff)
  pol1_in1_first_tap9/4(taps_out) -> pol1_in1_tap112/4(taps)
  pol1_in1_tap112/1(dout) -> pol1_in1_tap111/1(din)
  pol1_in1_tap112/2(sync_out) -> pol1_in1_tap111/2(sync)
  pol1_in1_tap112/3(coeff_out) -> pol1_in1_tap111/3(coeff)
  pol1_in1_tap112/4(taps_out) -> pol1_in1_tap111/4(taps)
  pol1_in1_tap111/1(dout) -> pol1_in1_tap58/1(din)
  pol1_in1_tap111/2(sync_out) -> pol1_in1_tap58/2(sync)
  pol1_in1_tap111/3(coeff_out) -> pol1_in1_tap58/3(coeff)
  pol1_in1_tap111/4(taps_out) -> pol1_in1_tap58/4(taps)
  pol1_in1_tap58/1(dout) -> pol1_in1_tap59/1(din)
  pol1_in1_tap58/2(sync_out) -> pol1_in1_tap59/2(sync)
  pol1_in1_tap58/3(coeff_out) -> pol1_in1_tap59/3(coeff)
  pol1_in1_tap58/4(taps_out) -> pol1_in1_tap59/4(taps)
  pol1_in1_tap59/1(dout) -> pol1_in1_tap60/1(din)
  pol1_in1_tap59/2(sync_out) -> pol1_in1_tap60/2(sync)
  pol1_in1_tap59/3(coeff_out) -> pol1_in1_tap60/3(coeff)
  pol1_in1_tap59/4(taps_out) -> pol1_in1_tap60/4(taps)
  pol1_in1_tap60/1(dout) -> pol1_in1_tap61/1(din)
  pol1_in1_tap60/2(sync_out) -> pol1_in1_tap61/2(sync)
  pol1_in1_tap60/3(coeff_out) -> pol1_in1_tap61/3(coeff)
  pol1_in1_tap60/4(taps_out) -> pol1_in1_tap61/4(taps)
  pol1_in1_tap61/1(dout) -> pol1_in1_tap62/1(din)
  pol1_in1_tap61/2(sync_out) -> pol1_in1_tap62/2(sync)
  pol1_in1_tap61/3(coeff_out) -> pol1_in1_tap62/3(coeff)
  pol1_in1_tap61/4(taps_out) -> pol1_in1_tap62/4(taps)
  pol1_in1_tap109/4(taps_out) -> pol1_in1_tap110/4(taps)
  pol1_in1_tap109/3(coeff_out) -> pol1_in1_tap110/3(coeff)
  pol1_in1_tap109/2(sync_out) -> pol1_in1_tap110/2(sync)
  pol1_in1_tap109/1(dout) -> pol1_in1_tap110/1(din)
  pol1_in1_tap101/4(taps_out) -> pol1_in1_tap109/4(taps)
  pol1_in1_tap101/3(coeff_out) -> pol1_in1_tap109/3(coeff)
  pol1_in1_tap101/2(sync_out) -> pol1_in1_tap109/2(sync)
  pol1_in1_tap101/1(dout) -> pol1_in1_tap109/1(din)
  pol1_in1_tap90/4(taps_out) -> pol1_in1_tap101/4(taps)
  pol1_in1_tap90/3(coeff_out) -> pol1_in1_tap101/3(coeff)
  pol1_in1_tap90/2(sync_out) -> pol1_in1_tap101/2(sync)
  pol1_in1_tap90/1(dout) -> pol1_in1_tap101/1(din)
  pol1_in1_tap79/4(taps_out) -> pol1_in1_tap90/4(taps)
  pol1_in1_tap79/3(coeff_out) -> pol1_in1_tap90/3(coeff)
  pol1_in1_tap79/2(sync_out) -> pol1_in1_tap90/2(sync)
  pol1_in1_tap79/1(dout) -> pol1_in1_tap90/1(din)
  pol1_in1_tap57/4(taps_out) -> pol1_in1_tap79/4(taps)
  pol1_in1_tap57/3(coeff_out) -> pol1_in1_tap79/3(coeff)
  pol1_in1_tap57/2(sync_out) -> pol1_in1_tap79/2(sync)
  pol1_in1_tap57/1(dout) -> pol1_in1_tap79/1(din)
  pol1_in1_tap68/4(taps_out) -> pol1_in1_tap57/4(taps)
  pol1_in1_tap68/3(coeff_out) -> pol1_in1_tap57/3(coeff)
  pol1_in1_tap68/2(sync_out) -> pol1_in1_tap57/2(sync)
  pol1_in1_tap68/1(dout) -> pol1_in1_tap57/1(din)
  pol1_in1_first_tap8/4(taps_out) -> pol1_in1_tap68/4(taps)
  pol1_in1_first_tap8/3(coeff_out) -> pol1_in1_tap68/3(coeff)
  pol1_in1_first_tap8/2(sync_out) -> pol1_in1_tap68/2(sync)
  pol1_in1_first_tap8/1(dout) -> pol1_in1_tap68/1(din)
  pol1_in1_tap14/2(taps_out) -> pol1_out2/1
  pol1_in1_tap19/2(taps_out) -> pol1_out3/1
  pol1_in1_tap26/2(taps_out) -> pol1_out4/1
  pol1_in1_tap54/2(taps_out) -> pol1_out5/1
  pol1_in1_tap34/2(taps_out) -> pol1_out6/1
  pol1_in1_tap39/2(taps_out) -> pol1_out7/1
  pol1_in1_tap47/2(taps_out) -> pol1_out8/1
  pol1_in1_tap110/2(taps_out) -> pol2_out1/1
  pol1_in1_tap62/2(taps_out) -> pol2_out2/1
  pol1_in1_tap67/2(taps_out) -> pol2_out3/1
  pol1_in1_tap75/2(taps_out) -> pol2_out4/1
  pol1_in1_tap106/2(taps_out) -> pol2_out5/1
  pol1_in1_tap84/2(taps_out) -> pol2_out6/1
  pol1_in1_tap89/2(taps_out) -> pol2_out7/1
  pol1_in1_tap98/2(taps_out) -> pol2_out8/1
